simulink model "Complete_Model"  (R2023a)
settings: Solver=VariableStepAuto  StartTime=0.0  StopTime=22  MinStep=5e-5  RelTol=1e-3
values from neighbouring files (symbols this model uses):
  Cbus = 80e-3*n_wt_agg*u^2    (= 1.33333333333)    [param.m]
  D = 1e-3    (= 0.001)    [param.m]
  f = 50    [param.m]
  Fs = Vs * sqrt(2/3)/(2*pi*f)    [param.m]
  fsw = 4e3    (= 4000)    [param.m]
  Idr_rated = sqrt(2)*Is    [param.m]
  J = 127*n_wt_agg    (= 19050)    [param.m]
  ki_id = (wni^2)*Lr*sigma    (= 3.27733001332)    [param.m]
  ki_idg = (wnig^2)*Lg    (= 0.263189450696)    [param.m]
  ki_iq = ki_id    (= 3.27733001332)    [param.m]
  ki_iqg = ki_idg    (= 0.263189450696)    [param.m]
  ki_v = -300000*n_wt_agg    (= -45000000)    [param.m]
  Kopt = ((0.5*rho*pi*(Radius^5)*Cp_max)/(lambda_opt^3))    (= 296454.198186)    [param.m]
  kp_id = (2*wni*sigma*Lr) - Rr    (= 0.00384733333333)    [param.m]
  kp_idg = ((2*wnig*Lg)-Rg)    (= 0.00167538274858)    [param.m]
  kp_iq = kp_id    (= 0.00384733333333)    [param.m]
  kp_iqg = kp_idg    (= 0.00167538274858)    [param.m]
  kp_v = -1000*n_wt_agg    (= -150000)    [param.m]
  Kpg = 1/(1.5*Vr*sqrt(2/3))    [param.m]
  Kqg = -Kpg    [param.m]
  lambda_opt = 7.2    [param.m]
  Lg = 400e-6 /n_wt_agg    (= 2.66666666667e-06)    [param.m]
  Lm = 2.5e-3 /n_wt_agg    (= 1.66666666667e-05)    [param.m]
  Lr = Lm + Lsi    (= 1.72466666667e-05)    [param.m]
  Ls = Lm + Lsi    (= 1.72466666667e-05)    [param.m]
  Lsi = 0.087e-3 /n_wt_agg    (= 5.8e-07)    [param.m]
  N = 100    [param.m]
  n_wt_agg = 150    [param.m]
  p = 2    [param.m]
  Ps = 2e6*n_wt_agg    (= 300000000)    [param.m]
  Radius = 42    [param.m]
  Rg = 20e-6 /n_wt_agg    (= 1.33333333333e-07)    [param.m]
  rho = 1.225    [param.m]
  Rr = 2.9e-3 /n_wt_agg    (= 1.93333333333e-05)    [param.m]
  Rs = 2.6e-3 /n_wt_agg    (= 1.73333333333e-05)    [param.m]
  sigma = 1 - Lm^2 / (Ls*Lr)    (= 0.0661284179513)    [param.m]
  smax = 1/3    (= 0.333333333333)    [param.m]
  tab_lambda = [0.1:0.01:11.8]    [param.m]
  Vbus = 1150/u    (= 3450)    [param.m]
  Vs = 690    [param.m]
undefined symbols (not defined in the file or in its folder): Cp, D1, Delta, DiscIntNeverNeededParam, magnitude
libraries: spsSecondOrderFilterLib x133, spsThreePhaseVIMeasurementLib x58, spsPower3phInstantaneousLib x52, simulink x41, spsThreePhaseTransformerTwoWindingsLib x27, spsUniversalBridgeLib x22, spsPWMGenerator2LevelLib x22, spsSeriesRLCBranchLib x19, spsFirstOrderFilterLib x18, spsThreePhasePISectionLineLib x17, spsGroundLib x17, spsThreePhaseSeriesRLCBranchLib x17
(part 8 of 15)

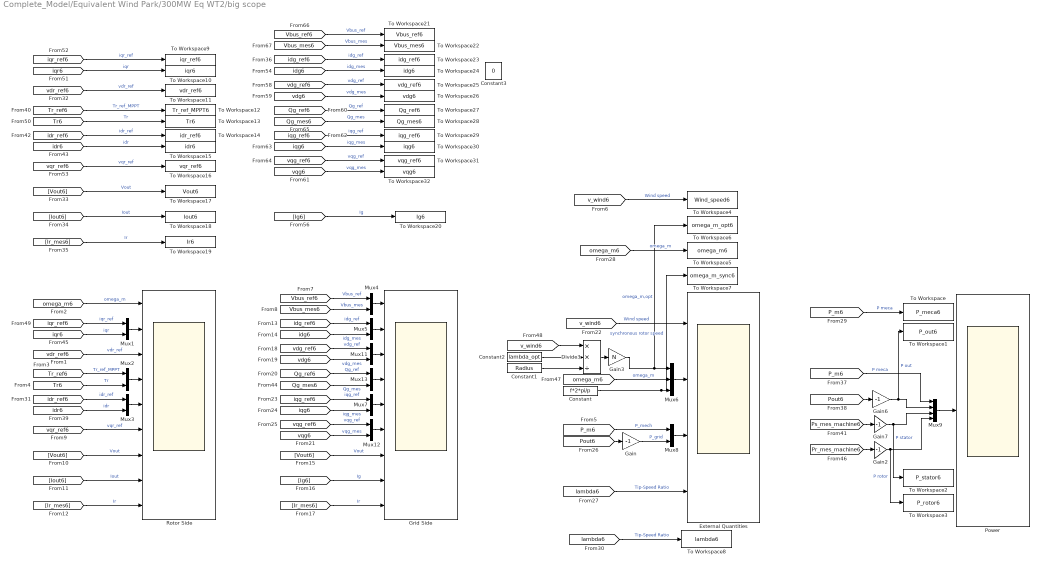
== system Complete_Model/Equivalent Wind Park/300MW Eq WT2/big scope ==
  Constant : Constant  Value=f*2*pi/p
  Constant1 : Constant  Value=Radius
  Constant2 : Constant  Value=lambda_opt
  Constant3 : Constant  Value=0
  Divide3 : Product  Inputs=**/
  External Quantities : Scope  NumInputPorts=4
  Gain{,2,6..7} (4 alike) : Gain  Gain=-1
  Gain3 : Gain  Gain=N
  Grid Side : Scope  NumInputPorts=9
  Mux{1..5,7..8,11..13} (10 alike) : Mux  Inputs=2
  Mux6 : Mux  Inputs=3
  Mux9 : Mux
  Power : Scope
  Rotor Side : Scope  NumInputPorts=9
  To Workspace : ToWorkspace  VariableName=P_meca6  SaveFormat=Timeseries  SampleTime=-1
  To Workspace1 : ToWorkspace  VariableName=P_out6  SaveFormat=Timeseries  SampleTime=-1
  To Workspace10 : ToWorkspace  VariableName=iqr6  SaveFormat=Timeseries  SampleTime=-1
  To Workspace11 : ToWorkspace  VariableName=vdr_ref6  SaveFormat=Timeseries  SampleTime=-1
  To Workspace12 : ToWorkspace  VariableName=Tr_ref_MPPT6  SaveFormat=Timeseries  SampleTime=-1
  To Workspace13 : ToWorkspace  VariableName=Tr6  SaveFormat=Timeseries  SampleTime=-1
  To Workspace14 : ToWorkspace  VariableName=idr_ref6  SaveFormat=Timeseries  SampleTime=-1
  To Workspace15 : ToWorkspace  VariableName=idr6  SaveFormat=Timeseries  SampleTime=-1
  To Workspace16 : ToWorkspace  VariableName=vqr_ref6  SaveFormat=Timeseries  SampleTime=-1
  To Workspace17 : ToWorkspace  VariableName=Vout6  SaveFormat=Timeseries  SampleTime=-1
  To Workspace18 : ToWorkspace  VariableName=Iout6  SaveFormat=Timeseries  SampleTime=-1
  To Workspace19 : ToWorkspace  VariableName=Ir6  SaveFormat=Timeseries  SampleTime=-1
  To Workspace2 : ToWorkspace  VariableName=P_stator6  SaveFormat=Timeseries  SampleTime=-1
  To Workspace20 : ToWorkspace  VariableName=Ig6  SaveFormat=Timeseries  SampleTime=-1
  To Workspace21 : ToWorkspace  VariableName=Vbus_ref6  SaveFormat=Timeseries  SampleTime=-1
  To Workspace22 : ToWorkspace  VariableName=Vbus_mes6  SaveFormat=Timeseries  SampleTime=-1
  To Workspace23 : ToWorkspace  VariableName=idg_ref6  SaveFormat=Timeseries  SampleTime=-1
  To Workspace24 : ToWorkspace  VariableName=idg6  SaveFormat=Timeseries  SampleTime=-1
  To Workspace25 : ToWorkspace  VariableName=vdg_ref6  SaveFormat=Timeseries  SampleTime=-1
  To Workspace26 : ToWorkspace  VariableName=vdg6  SaveFormat=Timeseries  SampleTime=-1
  To Workspace27 : ToWorkspace  VariableName=Qg_ref6  SaveFormat=Timeseries  SampleTime=-1
  To Workspace28 : ToWorkspace  VariableName=Qg_mes6  SaveFormat=Timeseries  SampleTime=-1
  To Workspace29 : ToWorkspace  VariableName=iqg_ref6  SaveFormat=Timeseries  SampleTime=-1
  To Workspace3 : ToWorkspace  VariableName=P_rotor6  SaveFormat=Timeseries  SampleTime=-1
  To Workspace30 : ToWorkspace  VariableName=iqg6  SaveFormat=Timeseries  SampleTime=-1
  To Workspace31 : ToWorkspace  VariableName=vqg_ref6  SaveFormat=Timeseries  SampleTime=-1
  To Workspace32 : ToWorkspace  VariableName=vqg6  SaveFormat=Timeseries  SampleTime=-1
  To Workspace4 : ToWorkspace  VariableName=Wind_speed6  SaveFormat=Timeseries  SampleTime=-1
  To Workspace5 : ToWorkspace  VariableName=omega_m6  SaveFormat=Timeseries  SampleTime=-1
  To Workspace6 : ToWorkspace  VariableName=omega_m_opt6  SaveFormat=Timeseries  SampleTime=-1
  To Workspace7 : ToWorkspace  VariableName=omega_m_sync6  SaveFormat=Timeseries  SampleTime=-1
  To Workspace8 : ToWorkspace  VariableName=lambda6  SaveFormat=Timeseries  SampleTime=-1
  To Workspace9 : ToWorkspace  VariableName=iqr_ref6  SaveFormat=Timeseries  SampleTime=-1
  wires:
  [vqg6] -> To Workspace32/1  "vqg_mes"
  [vqg_ref6] -> To Workspace31/1  "vqg_ref"
  [iqg6] -> To Workspace30/1  "iqg_mes"
  [iqg_ref6] -> To Workspace29/1  "iqg_ref"
  [Qg_mes6] -> To Workspace28/1  "Qg_mes"
  [vdg6] -> To Workspace26/1  "vdg_mes"
  [Qg_ref6] -> To Workspace27/1  "Qg_ref"
  [vdg_ref6] -> To Workspace25/1  "vdg_ref"
  [idg6] -> To Workspace24/1  "idg_mes"
  [idg_ref6] -> To Workspace23/1  "idg_ref"
  [Vbus_mes6] -> To Workspace22/1  "Vbus_mes"
  [Vbus_ref6] -> To Workspace21/1  "Vbus_ref"
  [Ig6] -> To Workspace20/1  "Ig"
  [Ir_mes6] -> To Workspace19/1  "Ir"
  [Iout6] -> To Workspace18/1  "Iout"
  [Vout6] -> To Workspace17/1  "Vout"
  [vqr_ref6] -> To Workspace16/1  "vqr_ref"
  [idr6] -> To Workspace15/1  "idr"
  [idr_ref6] -> To Workspace14/1  "idr_ref"
  [Tr6] -> To Workspace13/1  "Tr"
  [Tr_ref6] -> To Workspace12/1  "Tr_ref_MPPT"
  [vdr_ref6] -> To Workspace11/1  "vdr_ref"
  [iqr6] -> To Workspace10/1  "iqr"
  [iqr_ref6] -> To Workspace9/1  "iqr_ref"
  [lambda6] -> To Workspace8/1  "Tip-Speed Ratio"
  [P_m6] -> To Workspace/1  "P meca"
  [omega_m6] -> To Workspace5/1  "omega_m"
  [v_wind6] -> To Workspace4/1  "Wind speed"
  [lambda6] -> External Quantities/4  "Tip-Speed Ratio"
  Gain3/1 -> Mux6/1, To Workspace6/1  "omega_m,opt"
  Divide3/1 -> Gain3/1
  Constant1/1 -> Divide3/3
  Constant2/1 -> Divide3/2
  [v_wind6] -> Divide3/1
  [idr6] -> Mux3/2  "idr"
  [Tr6] -> Mux2/2  "Tr"
  [vdr_ref6] -> Rotor Side/3  "vdr_ref"
  [iqr_ref6] -> Mux1/1  "iqr_ref"
  Mux13/1 -> Grid Side/4
  [Qg_mes6] -> Mux13/2  "Qg_mes"
  [Qg_ref6] -> Mux13/1  "Qg_ref"
  Mux12/1 -> Grid Side/6
  [vqg_ref6] -> Mux12/1  "vqg_ref"
  [vqg6] -> Mux12/2  "vqg_mes"
  Gain2/1 -> Mux9/4, To Workspace3/1  "P rotor"
  [Pout6] -> Gain6/1
  Mux9/1 -> Power/1
  [P_m6] -> Mux9/1  "P meca"
  [Pr_mes_machine6] -> Gain2/1
  Gain7/1 -> Mux9/3, To Workspace2/1  "P stator"
  [Ps_mes_machine6] -> Gain7/1
  Gain6/1 -> Mux9/2, To Workspace1/1  "P out"
  Mux11/1 -> Grid Side/3
  [vdg6] -> Mux11/2  "vdg_mes"
  [vdg_ref6] -> Mux11/1  "vdg_ref"
  [P_m6] -> Mux8/1  "P_mech"
  Constant/1 -> Mux6/3, To Workspace7/1  "synchronous rotor speed"
  [omega_m6] -> Mux6/2  "omega_m"
  [v_wind6] -> External Quantities/1  "Wind speed"
  Mux6/1 -> External Quantities/2
  Mux8/1 -> External Quantities/3
  [Pout6] -> Gain/1
  Gain/1 -> Mux8/2  "P_grid"
  [omega_m6] -> Rotor Side/1  "omega_m"
  [idg_ref6] -> Mux5/1  "idg_ref"
  Mux4/1 -> Grid Side/1
  [idg6] -> Mux5/2  "idg_mes"
  Mux5/1 -> Grid Side/2
  [Vbus_mes6] -> Mux4/2  "Vbus_mes"
  Mux7/1 -> Grid Side/5
  [Vbus_ref6] -> Mux4/1  "Vbus_ref"
  [iqg6] -> Mux7/2  "iqg_mes"
  [Ir_mes6] -> Grid Side/9  "Ir"
  [Vout6] -> Grid Side/7  "Vout"
  [iqg_ref6] -> Mux7/1  "iqg_ref"
  [Ig6] -> Grid Side/8  "Ig"
  [Ir_mes6] -> Rotor Side/9  "Ir"
  [Iout6] -> Rotor Side/8  "Iout"
  [Vout6] -> Rotor Side/7  "Vout"
  [vqr_ref6] -> Rotor Side/6  "vqr_ref"
  [idr_ref6] -> Mux3/1  "idr_ref"
  Mux3/1 -> Rotor Side/5
  Mux2/1 -> Rotor Side/4
  [Tr_ref6] -> Mux2/1  "Tr_ref_MPPT"
  Mux1/1 -> Rotor Side/2
  [iqr6] -> Mux1/2  "iqr"

== system Complete_Model/Equivalent Wind Park/300MW Eq WT3/Aerodynamic Model ==
  note: Gearbox ratio
  note: Motor convention : Generated power
      |  is negative
  note: \Omega_m blades
  note: Cp
  note: \lambda = \Omega_m R / v_{wind}
  note: C_p (\lambda)
  note: v_{wind}
  Vw : Inport
  C_p (\lambda) : Lookup_n-D  NumberOfTableDimensions=1  BreakpointsForDimension1=tab_lambda  Table=Cp  InternalRulePriority=Speed  RndMeth=Simplest
  Constant : Constant  Value=Radius
  Divide : Product  Inputs=*/
  Divide1 : Product  Inputs=**/
  Fcn2 : Fcn  Expr=0.5*rho*pi*Radius^2*u(2)^3*u(1)
  Gain : Gain  Gain=1/N
  Gain2 : Gain  Gain=n_wt_agg
  Gain4 : Gain  Gain=-1
  Mux2 : Mux  Inputs=2
  Tm : Outport
  tag scope: P_m10=global, Tm10=global, lambda10=global
  wires:
  Gain4/1 -> Divide/1
  Constant/1 -> Divide1/1
  Divide/1 -> Tm/1, [Tm10]
  [omega_m10] -> Divide/2
  Gain2/1 -> Gain4/1, [P_m10]
  Fcn2/1 -> Gain2/1
  Mux2/1 -> Fcn2/1
  C_p (\lambda)/1 -> Mux2/1
  Divide1/1 -> [lambda10], C_p (\lambda)/1  "lambda"
  Vw/1 -> Mux2/2, Divide1/3
  Gain/1 -> Divide1/2
  [omega_m10] -> Gain/1

== system Complete_Model/Equivalent Wind Park/300MW Eq WT3/Grid Side Control ==
  Vbus_ref : Inport
  Qg_ref : Inport  Port=2
  Vbus : Inport  Port=3
  Ig_mes : Inport  Port=4
  Vs_mes : Inport  Port=5
  Demux : Demux  Outputs=2
  Demux1 : Demux  Outputs=2
  Gain : Gain  Gain=Kqg
  Gain1 : Gain  Gain=Kpg
  Normalized for PWM : Gain  Gain=1/(Vbus/2)
  "PI " : Subsystem
  PI id : Subsystem
  PI iq : Subsystem
  Sum : Sum  Inputs=|++
  Vs_mes1 : InportShadow  Port=5
  abc->alpha/beta : Subsystem
  abc->alpha/beta1 : Subsystem
  alpha/beta->abc : Subsystem
  alpha/beta->d/q : Subsystem
  alpha/beta->d/q1 : Subsystem
  angle2 : Subsystem
  cancellation of coupling terms : Subsystem
  d/q->alpha/beta : Subsystem
  Vabc_ref : Outport
  tag scope: Qg_ref10=global, Vbus_ref10=global, idg10=global, idg_ref10=global, iqg10=global, iqg_ref10=global, vdg10=global, vqg10=global
  wires:
  Vs_mes1/1 -> Sum/2
  angle2/1(theta_g) -> [theta_grid10]
  [theta_grid10] -> alpha/beta->d/q1/2(theta)
  [theta_grid10] -> d/q->alpha/beta/2(theta)
  [theta_grid10] -> alpha/beta->d/q/2(theta)
  Demux/2 -> PI iq/2(meas), [iqg10]
  Demux/1 -> PI id/2(meas), [idg10]
  Vbus/1 -> "PI "/2(meas)
  Demux1/1 -> [vdg10]
  Demux1/2 -> [vqg10]
  alpha/beta->d/q1/1(d/q) -> Demux1/1
  abc->alpha/beta1/1(alpha/beta) -> alpha/beta->d/q1/1(D/Q)
  [Vg10] -> abc->alpha/beta1/1(abc)
  cancellation of coupling terms/1(out) -> d/q->alpha/beta/1(d/q)
  Sum/1 -> Normalized for PWM/1
  alpha/beta->abc/1(abc) -> Sum/1
  Qg_ref/1 -> Gain/1, [Qg_ref10]
  Gain1/1 -> PI id/1(ref), [idg_ref10]  "idg"
  "PI "/1(out) -> Gain1/1  "Pg_ref"
  Gain/1 -> [iqg_ref10], PI iq/1(ref)  "iqg"
  Vbus_ref/1 -> "PI "/1(ref), [Vbus_ref10]
  alpha/beta->d/q/1(d/q) -> Demux/1
  PI iq/1(out) -> cancellation of coupling terms/2(Vq*)
  PI id/1(out) -> cancellation of coupling terms/1(Vd*)
  Vs_mes/1 -> angle2/1(Vs_mes)
  Normalized for PWM/1 -> Vabc_ref/1
  abc->alpha/beta/1(alpha/beta) -> alpha/beta->d/q/1(D/Q)
  Ig_mes/1 -> abc->alpha/beta/1(abc)
  d/q->alpha/beta/1(D/Q) -> alpha/beta->abc/1(D/Q)

== system Complete_Model/Equivalent Wind Park/300MW Eq WT3/Power Calculations ==
  Demux{,1..3} (4 alike) : Demux  Outputs=2
  Divide : Product  Inputs=**
  Gain : Gain  Gain=3/2
  Gain1 : Gain  Gain=-1
  Gain2 : Gain  Gain=-1
  Power (3ph, Instantaneous){1..4} (4 alike) : spsPower3phInstantaneousLib/Power (3ph, Instantaneous)
  Scope{1..8,10..13} (12 alike) : Scope
  Scope9 : Scope  NumInputPorts=2
  "Second-Order " : spsSecondOrderFilterLib/Second-Order Filter  FilterType=Lowpass  Fo=17  Zeta=0.7  Ts=7.4074e-05  Initialize=off  Vac_Init="[0,  0,  60]"  Vdc_Init=0  PlotResponse=off  FreqRange=[0, 500, 1]
      settings: AttributesFormatString=f0=%<Fo>
  Second-Order 1 : spsSecondOrderFilterLib/Second-Order Filter  FilterType=Lowpass  Fo=17  Zeta=0.7  Ts=7.4074e-05  Initialize=off  Vac_Init="[0,  0,  60]"  Vdc_Init=0  PlotResponse=off  FreqRange=[0, 500, 1]
      settings: AttributesFormatString=f0=%<Fo>
  Second-Order Low-Pass Filter {1..7} (7 alike) : spsSecondOrderFilterLib/Second-Order Filter  FilterType=Lowpass  Fo=17  Zeta=0.7  Ts=7.4074e-05  Initialize=off  Vac_Init="[0,  0,  60]"  Vdc_Init=0  PlotResponse=off  FreqRange=[0, 500, 1]
      settings: AttributesFormatString=f0=%<Fo>
  Subsystem : Subsystem
  Subsystem1 : Subsystem
  Subsystem2 : Subsystem
  Subsystem3 : Subsystem
  To Workspace1 : ToWorkspace  VariableName=Iout_d10  SaveFormat=Timeseries  SampleTime=-1
  To Workspace2 : ToWorkspace  VariableName=Ig_d10  SaveFormat=Timeseries  SampleTime=-1
  To Workspace21 : ToWorkspace  VariableName=Vout_d10  SaveFormat=Timeseries  SampleTime=-1
  To Workspace3 : ToWorkspace  VariableName=Vg_d10  SaveFormat=Timeseries  SampleTime=-1
  alpha/beta->d/q : Subsystem
  alpha/beta->d/q1 : Subsystem
  alpha/beta->d/q2 : Subsystem
  alpha/beta->d/q3 : Subsystem
  angle1 : Subsystem
  angle2 : Subsystem
  tag scope: Pg_mes10=global, Pout10=global, Pr_mes_machine10=global, Ps_mes_machine10=global, Qg_mes10=global, Qout10=global, Qr_mes_machine10=global, Qs_mes_machine10=global
  wires:
  [Vg10] -> angle1/1(Vs_mes), Subsystem2/1(abc)
  Subsystem2/1(alpha/beta) -> alpha/beta->d/q2/1(D/Q)
  alpha/beta->d/q2/1(d/q) -> Demux2/1
  Demux2/1 -> To Workspace3/1, Scope10/1
  Demux2/2 -> Scope11/1
  Demux3/2 -> Scope13/1
  Demux3/1 -> Scope12/1, To Workspace2/1
  alpha/beta->d/q3/1(d/q) -> Demux3/1
  Subsystem3/1(alpha/beta) -> alpha/beta->d/q3/1(D/Q)
  angle1/1(theta_g) -> alpha/beta->d/q3/2(theta), alpha/beta->d/q2/2(theta)
  [Ig10] -> Subsystem3/1(abc)
  Gain2/1 -> Second-Order 1/1
  Gain/1 -> Gain2/1
  Gain1/1 -> Scope9/2
  Second-Order 1/1 -> Scope9/1  "Pout"
  Divide/1 -> Gain/1
  [Iout10] -> Subsystem1/1(abc)
  angle2/1(theta_g) -> alpha/beta->d/q/2(theta), alpha/beta->d/q1/2(theta)
  Subsystem1/1(alpha/beta) -> alpha/beta->d/q1/1(D/Q)
  alpha/beta->d/q1/1(d/q) -> Demux1/1
  Demux1/1 -> To Workspace1/1, Scope7/1, Divide/2
  Demux1/2 -> Scope8/1
  Demux/2 -> Scope6/1
  Demux/1 -> Scope2/1, To Workspace21/1, Divide/1
  alpha/beta->d/q/1(d/q) -> Demux/1
  Subsystem/1(alpha/beta) -> alpha/beta->d/q/1(D/Q)
  [Vout10] -> Subsystem/1(abc), angle2/1(Vs_mes)
  [Vr_mes10] -> "Power (3ph, Instantaneous)4"/1
  "Power (3ph, Instantaneous)1"/1 -> Second-Order Low-Pass Filter 7/1  "Pr"
  "Power (3ph, Instantaneous)4"/1 -> Second-Order Low-Pass Filter 5/1  "Pr"
  [Vout10] -> "Power (3ph, Instantaneous)2"/1
  "Power (3ph, Instantaneous)3"/2 -> Second-Order Low-Pass Filter 2/1
  [Vg10] -> "Power (3ph, Instantaneous)1"/1
  Second-Order Low-Pass Filter 2/1 -> [Qs_mes_machine10]  "Q_stator"
  [Vs_mes_machine10] -> "Power (3ph, Instantaneous)3"/1
  "Power (3ph, Instantaneous)2"/1 -> "Second-Order "/1
  Second-Order Low-Pass Filter 5/1 -> [Pr_mes_machine10]  "P_rotor"
  [Is_mes_machine10] -> "Power (3ph, Instantaneous)3"/2, Scope4/1
  Second-Order Low-Pass Filter 7/1 -> [Pg_mes10]  "P_rotor"
  [Ir_mes10] -> "Power (3ph, Instantaneous)4"/2, Scope5/1
  "Second-Order "/1 -> [Pout10], Gain1/1  "Pout"
  Second-Order Low-Pass Filter 6/1 -> [Qg_mes10]  "Q_rotor"
  Second-Order Low-Pass Filter 4/1 -> [Qr_mes_machine10]  "Q_rotor"
  "Power (3ph, Instantaneous)3"/1 -> Second-Order Low-Pass Filter 3/1  "Ps"
  [Ig10] -> Scope1/1, "Power (3ph, Instantaneous)1"/2
  "Power (3ph, Instantaneous)4"/2 -> Second-Order Low-Pass Filter 4/1
  [Iout10] -> "Power (3ph, Instantaneous)2"/2, Scope3/1
  "Power (3ph, Instantaneous)1"/2 -> Second-Order Low-Pass Filter 6/1
  Second-Order Low-Pass Filter 3/1 -> [Ps_mes_machine10]  "P_stator"
  Second-Order Low-Pass Filter 1/1 -> [Qout10]  "Qout"
  "Power (3ph, Instantaneous)2"/2 -> Second-Order Low-Pass Filter 1/1

== system Complete_Model/Equivalent Wind Park/300MW Eq WT3/Rotor Side Control ==
  note: Stator referred
  idr_ref : Inport
  Ir_mes : Inport  Port=2
  Vs_mes : Inport  Port=3
  theta_m_mes : Inport  Port=4
  speed_mes : Inport  Port=5
  D/Q->abc : Subsystem
  D/Q->d/q : Subsystem
  Demux : Demux  Outputs=2
  Gain : Gain  Gain=1/(-1.5*p*(Lm/Ls)*Fs)
  Gain1 : Gain  Gain=1/u
  Gain2 : Gain  Gain=1/u
  MPPT : Subsystem
  Normalized for PWM : Gain  Gain=1/(Vbus/2)
  PI id : Subsystem
  PI iq : Subsystem
  abc->alpha/beta : Subsystem
  angle : Subsystem
  cancellation of coupling terms : Subsystem
  d/q->D/Q : Subsystem
  Vabc_ref : Outport
  tag scope: Tr_ref10=global, idr10=global, idr_ref10=global, iqr10=global, iqr_ref10=global, vrabc_ref10=global
  wires:
  MPPT/1(Tm,opt) -> Gain/1, [Tr_ref10]
  d/q->D/Q/1(D/Q) -> D/Q->abc/1(D/Q)
  abc->alpha/beta/1(alpha/beta) -> D/Q->d/q/1(D/Q)
  Gain2/1 -> [vrabc_ref10], Normalized for PWM/1  "Rotor referred"
  D/Q->abc/1(abc) -> Gain2/1
  Normalized for PWM/1 -> Vabc_ref/1
  Gain1/1 -> abc->alpha/beta/1(abc)
  idr_ref/1 -> PI id/1(ref), [idr_ref10]
  Gain/1 -> PI iq/1(ref), [iqr_ref10]
  Demux/2 -> PI iq/2(meas), [iqr10]
  Demux/1 -> [idr10], PI id/2(meas)
  D/Q->d/q/1(d/q) -> Demux/1  "i_dq = rotor ref frame"
  speed_mes/1 -> MPPT/1(omega_m), cancellation of coupling terms/3(omega_m)
  cancellation of coupling terms/1(out) -> d/q->D/Q/1(d/q)  "Vdr_ref,Vqr_ref"
  PI iq/1(out) -> cancellation of coupling terms/2(Vq*)
  PI id/1(out) -> cancellation of coupling terms/1(Vd*)
  angle/1(theta_r) -> D/Q->d/q/2(theta), d/q->D/Q/2(theta)
  Vs_mes/1 -> angle/1(Vs_mes)
  theta_m_mes/1 -> angle/2(theta_m)
  Ir_mes/1 -> Gain1/1

== system Complete_Model/Equivalent Wind Park/300MW Eq WT3/Subsystem ==
  In1 : Inport
  Bus Selector : BusSelector  OutputSignals=Mechanical.Rotor angle thetam (rad),Mechanical.Rotor speed (wm),Mechanical.Electromagnetic torque Te (N*m)
  Second-Order Low-Pass Filter 3 : spsSecondOrderFilterLib/Second-Order Filter  FilterType=Lowpass  Fo=17  Zeta=0.7  Ts=7.4074e-05  Initialize=off  Vac_Init="[0,  0,  60]"  Vdc_Init=0  PlotResponse=off  FreqRange=[0, 500, 1]
      settings: AttributesFormatString=f0=%<Fo>
  tag scope: Tr10=global, omega_m10=global, theta10=global
  wires:
  Second-Order Low-Pass Filter 3/1 -> [omega_m10]
  Bus Selector/3 -> [Tr10]  "<Electromagnetic torque Te (N*m)>"
  Bus Selector/1 -> [theta10]  "<Rotor angle thetam (rad)>"
  In1/1 -> Bus Selector/1
  Bus Selector/2 -> Second-Order Low-Pass Filter 3/1  "<Rotor speed (wm)>"

== system Complete_Model/Equivalent Wind Park/300MW Eq WT3/Subsystem1 ==
  Constant4 : Constant  Value=0
  Step : Step  Time=50  After=-0.2*Ps  SampleTime=0
  Sum14 : Sum  Inputs=++|
  Out1 : Outport
  wires:
  Sum14/1 -> Out1/1
  Step/1 -> Sum14/1
  Constant4/1 -> Sum14/2

== system Complete_Model/Equivalent Wind Park/300MW Eq WT3/Subsystem2 ==
  Constant1 : Constant  Value=9
  Ramp1 : simulink/Sources/Ramp  slope=-0.4  start=13  InitialOutput=0
  Ramp2 : simulink/Sources/Ramp  slope=0.4  start=8  InitialOutput=0
  Sum1 : Sum  Inputs=++|
  Sum2 : Sum  Inputs=++|
  v_wind : Outport
  tag scope: v_wind10=global
  wires:
  Constant1/1 -> Sum2/2
  Ramp2/1 -> Sum1/1
  Ramp1/1 -> Sum1/2
  Sum2/1 -> v_wind/1, [v_wind10]
  Sum1/1 -> Sum2/1

== system Complete_Model/Equivalent Wind Park/300MW Eq WT3/Subsystem8 ==
  Constant4 : Constant  Value=0
  Step : Step  Time=55  After=Idr_rated*0.2  SampleTime=0
  Sum14 : Sum  Inputs=++|
  Out1 : Outport
  wires:
  Sum14/1 -> Out1/1
  Constant4/1 -> Sum14/2
  Step/1 -> Sum14/1

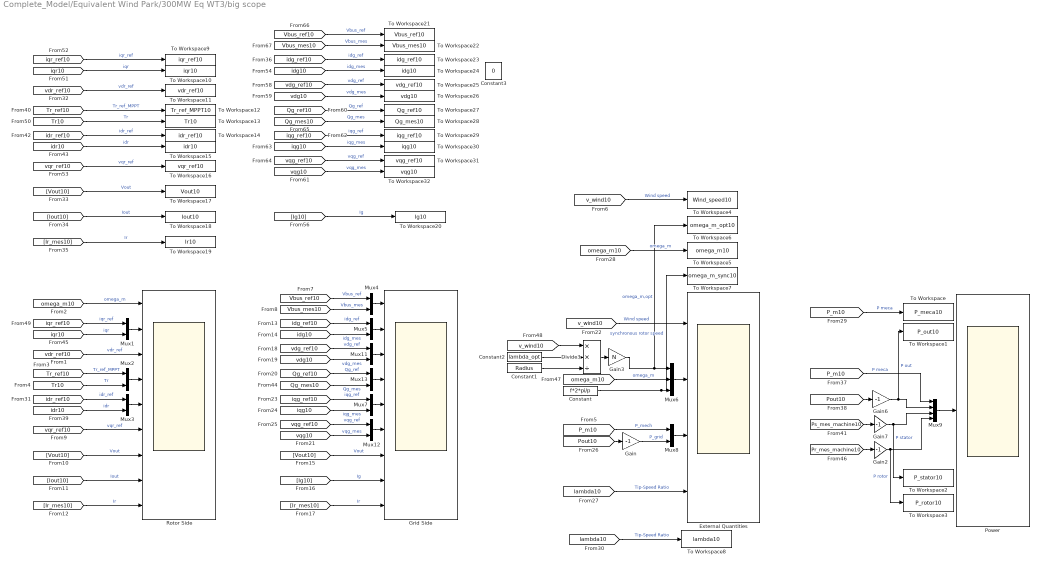
== system Complete_Model/Equivalent Wind Park/300MW Eq WT3/big scope ==
  Constant : Constant  Value=f*2*pi/p
  Constant1 : Constant  Value=Radius
  Constant2 : Constant  Value=lambda_opt
  Constant3 : Constant  Value=0
  Divide3 : Product  Inputs=**/
  External Quantities : Scope  NumInputPorts=4
  Gain{,2,6..7} (4 alike) : Gain  Gain=-1
  Gain3 : Gain  Gain=N
  Grid Side : Scope  NumInputPorts=9
  Mux{1..5,7..8,11..13} (10 alike) : Mux  Inputs=2
  Mux6 : Mux  Inputs=3
  Mux9 : Mux
  Power : Scope
  Rotor Side : Scope  NumInputPorts=9
  To Workspace : ToWorkspace  VariableName=P_meca10  SaveFormat=Timeseries  SampleTime=-1
  To Workspace1 : ToWorkspace  VariableName=P_out10  SaveFormat=Timeseries  SampleTime=-1
  To Workspace10 : ToWorkspace  VariableName=iqr10  SaveFormat=Timeseries  SampleTime=-1
  To Workspace11 : ToWorkspace  VariableName=vdr_ref10  SaveFormat=Timeseries  SampleTime=-1
  To Workspace12 : ToWorkspace  VariableName=Tr_ref_MPPT10  SaveFormat=Timeseries  SampleTime=-1
  To Workspace13 : ToWorkspace  VariableName=Tr10  SaveFormat=Timeseries  SampleTime=-1
  To Workspace14 : ToWorkspace  VariableName=idr_ref10  SaveFormat=Timeseries  SampleTime=-1
  To Workspace15 : ToWorkspace  VariableName=idr10  SaveFormat=Timeseries  SampleTime=-1
  To Workspace16 : ToWorkspace  VariableName=vqr_ref10  SaveFormat=Timeseries  SampleTime=-1
  To Workspace17 : ToWorkspace  VariableName=Vout10  SaveFormat=Timeseries  SampleTime=-1
  To Workspace18 : ToWorkspace  VariableName=Iout10  SaveFormat=Timeseries  SampleTime=-1
  To Workspace19 : ToWorkspace  VariableName=Ir10  SaveFormat=Timeseries  SampleTime=-1
  To Workspace2 : ToWorkspace  VariableName=P_stator10  SaveFormat=Timeseries  SampleTime=-1
  To Workspace20 : ToWorkspace  VariableName=Ig10  SaveFormat=Timeseries  SampleTime=-1
  To Workspace21 : ToWorkspace  VariableName=Vbus_ref10  SaveFormat=Timeseries  SampleTime=-1
  To Workspace22 : ToWorkspace  VariableName=Vbus_mes10  SaveFormat=Timeseries  SampleTime=-1
  To Workspace23 : ToWorkspace  VariableName=idg_ref10  SaveFormat=Timeseries  SampleTime=-1
  To Workspace24 : ToWorkspace  VariableName=idg10  SaveFormat=Timeseries  SampleTime=-1
  To Workspace25 : ToWorkspace  VariableName=vdg_ref10  SaveFormat=Timeseries  SampleTime=-1
  To Workspace26 : ToWorkspace  VariableName=vdg10  SaveFormat=Timeseries  SampleTime=-1
  To Workspace27 : ToWorkspace  VariableName=Qg_ref10  SaveFormat=Timeseries  SampleTime=-1
  To Workspace28 : ToWorkspace  VariableName=Qg_mes10  SaveFormat=Timeseries  SampleTime=-1
  To Workspace29 : ToWorkspace  VariableName=iqg_ref10  SaveFormat=Timeseries  SampleTime=-1
  To Workspace3 : ToWorkspace  VariableName=P_rotor10  SaveFormat=Timeseries  SampleTime=-1
  To Workspace30 : ToWorkspace  VariableName=iqg10  SaveFormat=Timeseries  SampleTime=-1
  To Workspace31 : ToWorkspace  VariableName=vqg_ref10  SaveFormat=Timeseries  SampleTime=-1
  To Workspace32 : ToWorkspace  VariableName=vqg10  SaveFormat=Timeseries  SampleTime=-1